simulink model "BMSModel"  (R2024b)
settings: Solver=VariableStepAuto  StartTime=0.0  StopTime=+inf  RelTol=1e-3
undefined symbols (not defined in the file): balancingRelaxationTime, balancingTime, ChargerParRes, ChargerRes, ChargingCurrentRequest, DeltaVTargetMin, DrivingCurrentRqst, InverterParRes, InverterRes, OpenCircuitInjection, PassiveBalancingRes, ShortCircuitInjection, sigbuilder_block, SystemState, SystemState_t
libraries: fl_lib x325, nesl_utility x223, batteryecm_lib x30, ee_lib x30
(part 17 of 33)

== system BMSModel/Electric Vehicul Model/HV Battery/Battery Pack/Battery Model1/Battery_Model/Cell3 ==
  ShortFault : Inport  OutDataTypeStr=boolean
  OpenFault : Inport  Port=2  OutDataTypeStr=boolean
  Battery (Table-Based)7 : batteryecm_lib/Battery (Table-Based)  PartSpecification="{\"DatasetId\":\"Panasonic|NCR18650BD|simscape.battery.batteryTableBased\",\"DisplayName\":\"Panasonic:NCR18650BD\"}"  SOC_vec=[0 0.01 0.02 0.03 0.04 0.05 0.06 0.07 0.08 0.09 0.1 0.11 0.12 0.13 0.14 0.15 0.16 0.17 0.18 0.19 0.2 0.21 0.22 0.23 0.24 0.25 0.26 0.27 0.28 0.29 0.3 0.31 0.32 0.33 0.34 0.35 0.36 0.37 0.38 0.39 0.4 0.41 0.42 0.43 0.44 0.45 0.46 0.47 0.48 0.49 0.5 0.51 0.52 0.53 0.54 0.55 0.56 0.57 0.58 0.59 0.6 0.61 0.62 0.63 0.64 0.65 0.66 0.67 0.68 0.69 0.7 0.71 0.72 0.73 0.74 0.75 0.76 0.77 0.78 0.79 0.8 0.81 0.82 0.83 0.84 0.85 0.86 0.87 0.88 0.89 0.9 0.91 0.92 0.93 0.94 0.95 0.96 0.97 0.98 0.99 1]  T_dependence=simscape.enum.tablebattery.temperature_dependence.yes  prm_dir=simscape.enum.tablebattery.prm_dir.noCurrentDirectionality  T_vec=[-10 0 25 40 60] degC  V0_mat=[3.01162183959268 3.01162183959268 3.01162183959268 3.01162183959268 3.01162183959268;3.08575650269622 3.08575650269622 3.08575650269622 3.08575650269622 3.08575650269622;3.14177499163339 3.14177499163339 3.14177499163339 3.14177499163339 3.14177499163339;3.18631823552003 3.18631823552003 3.18631823552003 3.18631823552003 3.18631823552003;3.22334954767724 3.22334954767724 3.22334954767724 3.22334954767724 3.22334954767724;3.25390136234099 3.25390136234099 3.25390136234099 3.25390136234099 3.25390136234099;3.27878869705109 3.27878869705109 3.27878869705109 3.27878869705109 3.27878869705109;3.30070350874259 3.30070350874259 3.30070350874259 3.30070350874259 3.30070350874259;3.32118523630503 3.32118523630503 3.32118523630503 3.32118523630503 3.32118523630503;3.33873222862982 3.33873222862982 3.33873222862982 3.33873222862982 3.33873222862982;3.35681278765286 3.35681278765286 3.35681278765286 3.35681278765286 3.35681278765286;3.3727103181478 3.3727103181478 3.3727103181478 3.3727103181478 3.3727103181478;3.38793252475269 3.38793252475269 3.38793252475269 3.38793252475269 3.38793252475269;3.40246133852855 3.40246133852855 3.40246133852855 3.40246133852855 3.40246133852855;3.41323877471787 3.41323877471787 3.41323877471787 3.41323877471787 3.41323877471787;3.42274053961048 3.42274053961048 3.42274053961048 3.42274053961048 3.42274053961048;3.43230513033606 3.43230513033606 3.43230513033606 3.43230513033606 3.43230513033606;3.44127066639713 3.44127066639713 3.44127066639713 3.44127066639713 3.44127066639713;3.45046092538443 3.45046092538443 3.45046092538443 3.45046092538443 3.45046092538443;3.46050021597496 3.46050021597496 3.46050021597496 3.46050021597496 3.46050021597496;3.47059444208772 3.47059444208772 3.47059444208772 3.47059444208772 3.47059444208772;3.47754582201769 3.47754582201769 3.47754582201769 3.47754582201769 3.47754582201769;3.48403887676592 3.48403887676592 3.48403887676592 3.48403887676592 3.48403887676592;3.49083263175798 3.49083263175798 3.49083263175798 3.49083263175798 3.49083263175798;3.49762638675004 3.49762638675004 3.49762638675004 3.49762638675004 3.49762638675004;3.50414427848956 3.50414427848956 3.50414427848956 3.50414427848956 3.50414427848956;3.51006189737342 3.51006189737342 3.51006189737342 3.51006189737342 3.51006189737342;3.51650779121088 3.51650779121088 3.51650779121088 3.51650779121088 3.51650779121088;3.52300031605604 3.52300031605604 3.52300031605604 3.52300031605604 3.52300031605604;3.5294928409012 3.5294928409012 3.5294928409012 3.5294928409012 3.5294928409012;3.53574150484027 3.53574150484027 3.53574150484027 3.53574150484027 3.53574150484027;3.54126505716736 3.54126505716736 3.54126505716736 3.54126505716736 3.54126505716736;3.54599126111241 3.54599126111241 3.54599126111241 3.54599126111241 3.54599126111241;3.55084210438451 3.55084210438451 3.55084210438451 3.55084210438451 3.55084210438451;3.55569196094666 3.55569196094666 3.55569196094666 3.55569196094666 3.55569196094666;3.56052372392349 3.56052372392349 3.56052372392349 3.56052372392349 3.56052372392349;3.56532059653064 3.56532059653064 3.56532059653064 3.56532059653064 3.56532059653064;3.56979945432131 3.56979945432131 3.56979945432131 3.56979945432131 3.56979945432131;3.57444121267711 3.57444121267711 3.57444121267711 3.57444121267711 3.57444121267711;3.57904303346503 3.57904303346503 3.57904303346503 3.57904303346503 3.57904303346503;3.58364485425295 3.58364485425295 3.58364485425295 3.58364485425295 3.58364485425295;3.58840361874736 3.58840361874736 3.58840361874736 3.58840361874736 3.58840361874736;3.5931676308725 3.5931676308725 3.5931676308725 3.5931676308725 3.5931676308725;3.59914045642067 3.59914045642067 3.59914045642067 3.59914045642067 3.59914045642067;3.60536834334135 3.60536834334135 3.60536834334135 3.60536834334135 3.60536834334135;3.61166009904557 3.61166009904557 3.61166009904557 3.61166009904557 3.61166009904557;3.61797346412467 3.61797346412467 3.61797346412467 3.61797346412467 3.61797346412467;3.62428682920377 ...(+4538 chars)] V  V0_vec=[3.01162183959268 3.08575650269622 3.14177499163339 3.18631823552003 3.22334954767724 3.25390136234099 3.27878869705109 3.30070350874259 3.32118523630503 3.33873222862982 3.35681278765286 3.3727103181478 3.38793252475269 3.40246133852855 3.41323877471787 3.42274053961048 3.43230513033606 3.44127066639713 3.45046092538443 3.46050021597496 3.47059444208772 3.47754582201769 3.48403887676592 3.49083263175798 3.49762638675004 3.50414427848956 3.51006189737342 3.51650779121088 3.52300031605604 3.5294928409012 3.53574150484027 3.54126505716736 3.54599126111241 3.55084210438451 3.55569196094666 3.56052372392349 3.56532059653064 3.56979945432131 3.57444121267711 3.57904303346503 3.58364485425295 3.58840361874736 3.5931676308725 3.59914045642067 3.60536834334135 3.61166009904557 3.61797346412467 3.62428682920377 3.63060019428287 3.63762035332317 3.64493091713641 3.65193784155324 3.65894476597006 3.66595169038689 3.67346631195551 3.68205597286888 3.69093403907072 3.69981210527255 3.70876940774879 3.71776352136614 3.72686785650522 3.73538877817079 3.74389655120326 3.7524031927436 3.76046489817023 3.76888762106825 3.7779024493972 3.78684332393235 3.79578419846749 3.80453642296868 3.81235798589345 3.82032795054978 3.82829600796219 3.83626406537459 3.84436267545785 3.8525073311682 3.86057855536852 3.86864977956884 3.87672100376915 3.8849361052788 3.89310639828889 3.90173837211624 3.91095173577988 3.92144530461468 3.9324037776784 3.94373568413836 3.95558619350771 3.96743304428185 3.9792002957498 3.99089798499064 4.00334195302942 4.01527114968024 4.0272843604214 4.03909414300309 4.04916289177822 4.05870791988182 4.06775178122738 4.07651000374835 4.08584401267932 4.09484130348047 4.10452854006648] V  V_range=[0, inf] V  R0_mat=[0.169226664942506 0.167163906452554 0.108383589577359 0.166496905009762 0.167551697989061;0.170828416476837 0.165751444040606 0.104276452250662 0.121215639388209 0.0793876425776906;0.169530739150659 0.158468431589049 0.0975073630060558 0.0973078843610968 0.0561405476976999;0.164751308135469 0.151517246065733 0.0937501268832215 0.0836197941247371 0.0456130421507902;0.161623504322352 0.145871801527593 0.0893819468578971 0.0721103823007927 0.0431619821725486;0.158185801688513 0.140686706979324 0.0854644713676261 0.0704282437680971 0.0421646037454825;0.154847008399576 0.135091432531267 0.08175755280038 0.0668766399041617 0.0396839944792877;0.151241736581272 0.129916346607537 0.0789657156023764 0.0623440053410213 0.0423144995660151;0.149319253943555 0.125715735974038 0.0763700410896046 0.0625490036389012 0.0444720395936461;0.147647439390094 0.12097131496664 0.074350005039498 0.0625868128655119 0.0460556489919985;0.146951059448331 0.118987805825618 0.0728433546029721 0.0628007167120074 0.0489709240971276;0.145717636926329 0.116283825223175 0.0716898267238281 0.0622941490970829 0.0511657277408373;0.144477070611268 0.114422945778514 0.0709947524858036 0.062440194574166 0.0531376522129154;0.143676208839892 0.113080299822462 0.0701675330798177 0.0632439744628587 0.0547714142497681;0.14163726865712 0.110499575455016 0.0687296636498536 0.0628096759401558 0.0549419374047933;0.139177314845068 0.107543098246653 0.0673712307537146 0.0619543637881734 0.0546914469305389;0.137590624546326 0.106293961693032 0.0662158126567781 0.0611197862345337 0.0544616910546272;0.135941778352897 0.104847117329339 0.0650205672726794 0.0601287634630414 0.054034227368643;0.134367098141725 0.103474438947903 0.0638581443118004 0.0597901127221725 0.0535283975759592;0.13307262638046 0.102457131174024 0.0632667622677959 0.0597316704312107 0.0532367466498601;0.132132723422297 0.101972409844216 0.0628323749302967 0.0596913586756406 0.0529632262591526;0.131094168008448 0.100450445549793 0.0619127995096658 0.058613803955455 0.0516524629038298;0.129904350158379 0.0987772188191482 0.0610362875653994 0.0575678584365115 0.0504293845103144;0.128813773312875 0.0972032330930701 0.0603620203223803 0.0567011683159597 0.0497501063026246;0.127723196467371 0.0960864247779599 0.0596877530793612 0.0558344781954072 0.049070828094934;0.126541575644132 0.0955051141824603 0.0589554578065768 0.0548767440971203 0.0483005059095089;0.125161844967536 0.0947256937336041 0.0582121279560435 0.0537209001454771 0.0473320738707274;0.124441622691283 0.0941206214542552 0.0577538001128378 0.0530375970606514 0.0465379900014533;0.123787439191075 0.0935309389464247 0.0573051863053598 0.0528219943808646 0.0460644521538233;0.123133255690868 0.0931004014618328 0.0568565724978822 0.052606391701078 0.0457423549094267;0.122398590043435 0.0926174478301112 0.0563566623767882 0.0523103068740658 0.0453397755178046;0.121424613632972 0.0918951834353595 0.0557889650711299 0.0517749112840235 0.0446978853631523;0.12018748594131 0.0909097677594091 0.0551527157042778 0.0509763644127827 0.0437928439273016;0.118892784708147 0.0899654871748907 0.0546601636283966 0.0502189526329738 0.0429623528682206;0.117592838262263 0.0890660124715378 0.0541676729953156 0.0494654301282659 0.0426851077795709;0.116286920336072 0.0881633432998133 0.0536763090566326 0.0487947175037582 0.042401891210615;0.11496948743639 0.0872491591545975 0.0531776059040762 0.0481124899057588 0.0421071596681674;0.113547099151729 0.0862300196244025 0.0526119236645951 0.0473253069227807 0.0417074727407412;0.112178473429822 0.0852646426569612 0.0520712300263533 0.0465918865025558 0.0413615483760683;0.110796666992441 0.0842860849740457 0.0515036752143963 0.045845285366857 0.0410024432959212;0.109615209979064 0.0832959889625606 0.0509361204024386 0.0450986842311574 0.0401864650192761;0.108594985808955 0.0823524881802858 0.050358792646556 0.0444566840657222 0.0392008538728351;0.107576493530178 0.0814107192893429 0.0497811381187243 0.0441001730364305 0.0382169746177263 ...(+5407 chars)] Ohm  R0_vec=[0.108383589577359 0.104276452250662 0.0975073630060558 0.0937501268832215 0.0893819468578971 0.0854644713676261 0.08175755280038 0.0789657156023764 0.0763700410896046 0.074350005039498 0.0728433546029721 0.0716898267238281 0.0709947524858036 0.0701675330798177 0.0687296636498536 0.0673712307537146 0.0662158126567781 0.0650205672726794 0.0638581443118004 0.0632667622677959 0.0628323749302967 0.0619127995096658 0.0610362875653994 0.0603620203223803 0.0596877530793612 0.0589554578065768 0.0582121279560435 0.0577538001128378 0.0573051863053598 0.0568565724978822 0.0563566623767882 0.0557889650711299 0.0551527157042778 0.0546601636283966 0.0541676729953156 0.0536763090566326 0.0531776059040762 0.0526119236645951 0.0520712300263533 0.0515036752143963 0.0509361204024386 0.050358792646556 0.0497811381187243 0.0494179007317464 0.0491061102548996 0.0488178197046288 0.0485440631684005 0.0482703066321729 0.0479965500959444 0.04783814754709 0.0477067926990279 0.0473712163371039 0.0470356399751792 0.0467000636132553 0.046431066764779 0.0463210072143651 0.0462716139198204 0.0462222206252753 0.0462261199933534 0.0462489440940044 0.0463125045945071 0.046258596645442 0.0462019228721321 0.0461444880808494 0.0458836890808223 0.04572086669122 0.0456935258730667 0.0456707901856076 0.0456480544981478 0.0455856361429723 0.0453236882021849 0.0450859495122444 0.0448483295871051 0.0446107096619657 0.0444608967355151 0.0443618965142366 0.0442474499230246 0.0441330033318128 0.0440185567406007 0.0437935866730952 0.0434800673484185 0.0432636629093245 0.0431695542324038 0.0433145404237094 0.0436166512557128 0.0439115402259747 0.0443155176840517 0.0447170344474344 0.0451426585495349 0.0455784262459232 0.0461653340876939 0.046366693627792 0.0465478472510504 0.046677433375216 0.0460938543815088 0.0452312262852973 0.044216043184874 0.0431407757562075 0.0413658805730101 0.0384867561999347 0.0332622873381256] Ohm  R0_dis_mat=[0.03 0.015 0.002;0.04 0.017 0.008;0.039 0.012 0.006;0.027 0.013 0.021] % Parameter not set Ohm  R0_dis_vec=[0.015 0.017 0.012 0.013] % Parameter not set Ohm  R0_ch_mat=[0.03 0.015 0.002;0.04 0.017 0.008;0.039 0.012 0.006;0.027 0.013 0.021] % Parameter not set Ohm  R0_ch_vec=[0.015 0.017 0.012 0.013] % Parameter not set Ohm  AH=[2.68349638863606 2.78552026614936 3.03732766876938 3.13981189304305 3.15345135361432] hr*A  prm_leak=simscape.enum.tablebattery.prm_leak.disabled  Rleak_vec=[8000 7000 6000] % Parameter not set Ohm  Rleak=7000 % Parameter not set Ohm  extrapolation_option=simscape.enum.extrapolation.linear  SOC_port=simscape.enum.tablebattery.enable.yes  prm_dyn=simscape.enum.tablebattery.prm_dyn.off  R1_mat=[0.089 0.076 0.01;0.042 0.022 0.099;0.019 0.007 0.002;0.051 0.043 0.029] % Parameter not set Ohm  R1_vec=[0.076 0.022 0.007 0.043] % Parameter not set Ohm  tau1_mat=[44 148 235;93 110 1000;19 27 133;0.5 22 3] % Parameter not set s  tau1_vec=[148 110 27 22] % Parameter not set s  R2_mat=[0.014 0.382 0.407;0.028 0.006 0.007;0.014 0.007 0.006;0.333 0.956 0.912] % Parameter not set Ohm  R2_vec=[0.382 0.006 0.007 0.956] % Parameter not set Ohm  tau2_mat=[1 44 5644;11 24 506;2 14 330;3310 13419 30216] % Parameter not set s  tau2_vec=[44 24 14 13419] % Parameter not set s  R3_mat=[0.014 0.382 0.407;0.028 0.006 0.007;0.014 0.007 0.006;0.333 0.956 0.912] % Parameter not set Ohm  R3_vec=[0.382 0.006 0.007 0.956] % Parameter not set Ohm  tau3_mat=[1 44 5644;11 24 506;2 14 330;3310 13419 30216] % Parameter not set s  tau3_vec=[44 24 14 13419] % Parameter not set s  R4_mat=[0.014 0.382 0.407;0.028 0.006 0.007;0.014 0.007 0.006;0.333 0.956 0.912] % Parameter not set Ohm  R4_vec=[0.382 0.006 0.007 0.956] % Parameter not set Ohm  tau4_mat=[1 44 5644;11 24 506;2 14 330;3310 13419 30216] % Parameter not set s  tau4_vec=[44 24 14 13419] % Parameter not set s  R5_mat=[0.014 0.382 0.407;0.028 0.006 0.007;0.014 0.007 0.006;0.333 0.956 0.912] % Parameter not set Ohm  R5_vec=[0.382 0.006 0.007 0.956] % Parameter not set Ohm  tau5_mat=[1 44 5644;11 24 506;2 14 330;3310 13419 30216] % Parameter not set s  tau5_vec=[44 24 14 13419] % Parameter not set s  prm_fade=simscape.enum.tablebattery.prm_fade.tableN  N0=100 % Parameter not set  dV0=0 % Parameter not set  dR0=0 % Parameter not set  dAH=0 % Parameter not set  dRleak=0 % Parameter not set  dR1=0 % Parameter not set  dR2=0 % Parameter not set  dR3=0 % Parameter not set  dR4=0 % Parameter not set  dR5=0 % Parameter not set  N0vec=[1 5 9 13 17 21 25 29 33 37 41 45 49 53 57 61 65 69 73 77 81 85 89 93 97 101 105 109 113 117 121 125 129 133 137 141 145 149 153 157 161 165 169 173 177 181 185 189 193 197 201 205 209 213 217 221 225 229 233 237 241 245 249 253 257 261 265 269 273 277 281 285 289 293 297 301 305 309 313 317]  Tfadevec=[298.15 323.15] % Parameter not set K  dV0vec=[0 -0.202976020343784 -0.3923769038231 -0.473851165498915 -0.557677193828717 -0.641503222158497 -0.722694953681098 -0.78693536781202 -0.84923483782873 -0.91204079498593 -0.976372086795922 -1.04070337860591 -1.09651419150015 -1.13609671747983 -1.17677806371868 -1.21745940995752 -1.25814075619637 -1.29882210243524 -1.33950344867411 -1.38190179312493 -1.42481526081378 -1.46425241362882 -1.5010921128618 -1.53793181209476 -1.57477151132773 -1.61462185034629 -1.65783167194698 -1.70104149354772 -1.74425131514844 -1.78746113674918 -1.83079973190849 -1.87421420391064 -1.91824367282699 -1.96240597622001 -2.00656827961306 -2.05073058300608 -2.09489288639913 -2.13905518979217 -2.17980859972762 -2.21447670676208 -2.24914481379657 -2.28381292083105 -2.3184810278655 -2.35314913489998 -2.38781724193444 -2.42248534896891 -2.45715345600337 -2.49296850346706 -2.52769329927103 -2.561744919227 -2.59579653918297 -2.62984815913894 -2.66389977909491 -2.69795139905088 -2.73200301900684 -2.76605463896281 -2.79820594865531 -2.82900596880459 -2.85607497867955 -2.88314398855452 -2.9102129984295 -2.93728200830447 -2.96435101817944 -2.99142002805441 -3.01848903792938 -3.04532930155381 -3.07028784008487 -3.09529445491126 -3.12030106973765 -3.14530768456405 -3.17031429939046 -3.19532091421685 -3.22227871749008 -3.25169561441252 -3.28111251133495 -3.31052940825736 -3.3399463051798 -3.36956112962439 -3.39917701648019 -3.42879290333599]  dR0vec=[0;0;0;0;0;0;0;0;0;0;0;0;0;0;0;0;0;0;0;0;0;0;0;0;0;0;0;0;0;0;0;0;0;0;0;0;0;0;0;0;0;0;0;0;0;0;0;0;0;0;0;0;0;0;0;0;0;0;0;0;0;0;0;0;0;0;0;0;0;0;0;0;0;0;0;0;0;0;0;0] % Parameter not set  dAHvec=[0 -0.860019120059007 -1.71332421702079 -2.08194002639565 -2.45055583577056 -2.81917164514544 -3.18778745452034 -3.48884924256567 -3.78081482359822 -4.07278040463078 -4.36474598566332 -4.65671156669588 -4.91000721732096 -5.08494410055826 -5.25988098379556 -5.43481786703286 -5.60975475027016 -5.78469163350746 -5.95962851674477 -6.13456539998206 -6.30950228321938 -6.47026795816407 -6.62044510557951 -6.77062225299495 -6.92079940041038 -7.07097654782583 -7.22115369524125 -7.3713308426567 -7.52150799007212 -7.67168513748756 -7.8223098416152 -7.97319825819041 -8.12408667476563 -8.27497509134084 -8.42586350791606 -8.57675192449127 -8.72764034106648 -8.8785287576417 -9.01789522921441 -9.13717923376072 -9.25646323830702 -9.37574724285333 -9.49503124739961 -9.61431525194592 -9.73359925649221 -9.85288326103849 -9.9721672655848 -10.0914512701311 -10.2059878190689 -10.3183039593139 -10.430620099559 -10.542936239804 -10.655252380049 -10.7675685202941 -10.8798846605391 -10.9922008007841 -11.1045169410292 -11.2125891928512 -11.3075699375911 -11.402550682331 -11.4975314270709 -11.5925121718108 -11.6874929165507 -11.7824736612906 -11.8774544060305 -11.9716325174515 -12.058857953676 -12.1460833899004 -12.2333088261248 -12.3205342623493 -12.4077596985737 -12.4949851347981 -12.5890165007581 -12.6916254178083 -12.7942343348584 -12.8968432519086 -12.9994521689588 -13.102061086009 -13.2046700030592 -13.3072789201093]  dRleakvec=[0 0 0] % Parameter not set  dR1vec=[0 0 0] % Parameter not set  dR2vec=[0 0 0] % Parameter not set  dR3vec=[0 0 0] % Parameter not set  dR4vec=[0 0 0] % Parameter not set  dR5vec=[0 0 0] % Parameter not set  dV0mat=[0 0;0 0;0 0] % Parameter not set  dR0mat=[0 0;0 0;0 0] % Parameter not set  dAHmat=[0 0;0 0;0 0] % Parameter not set  dRleakmat=[0 0;0 0;0 0] % Parameter not set  dR1mat=[0 0;0 0;0 0] % Parameter not set  dR2mat=[0 0;0 0;0 0] % Parameter not set  dR3mat=[0 0;0 0;0 0] % Parameter not set  dR4mat=[0 0;0 0;0 0] % Parameter not set  dR5mat=[0 0;0 0;0 0] % Parameter not set  prm_age_modeling=simscape.enum.tablebattery.prm_age_modeling.equation  storage_dt_age_vec=0 % Parameter not set d  storage_T_age_vec=273 % Parameter not set K  prm_age_OCV=simscape.enum.tablebattery.prm_age_OCV.SOC  OCV_age=0.9 % Parameter not set  SOC_age=0.6 % Parameter not set  prm_age_resistance=simscape.enum.tablebattery.prm_age.disabled  OCV_scaling_age_resistance=2213400  OCV_offset_age_resistance=1632000  temp_exp_age_resistance=0.515833569 V  t_exp_age_resistance=0.75  T_age_vec_resistance=[273.15 298.15 323.15] K  dt_age_vec_resistance=[90 180 270 360] d  dR0_age_vec=[0 0 0] % Parameter not set  t_meas_dR0_age=100 % Parameter not set d  dR0_age_mat=[0 0 0;0 0 0;0 0 0;0 0 0] % Parameter not set  prm_age_capacity=simscape.enum.tablebattery.prm_age.disabled  OCV_scaling_age_capacity=1.5097e07  OCV_offset_age_capacity=8.3625e06  temp_exp_age_capacity=0.6011 V  t_exp_age_capacity=0.75  T_age_vec_capacity=[273.15, 298.15, 323.15] K  dt_age_vec_capacity=[0, 90, 180, 270] d  dAH_age_vec=[0, 0, 0]  t_meas_dAH_age=100 d  dAH_age_mat=[0, 0, 0; 0, 0, 0; 0, 0, 0; 0, 0, 0]  thermal_port=simscape.enum.thermaleffects.model  temperature=298.15 K  thermal_mass=CellThermalMass J/K  i_specify=off  i_priority=None  i=0 A  i_nominal_specify=off  i_nominal_value=1  i_nominal_unit=A  v_specify=off  v_priority=None  v=0 V  v_nominal_specify=off  v_nominal_value=1  v_nominal_unit=V  stateOfCharge_specify=on  stateOfCharge_priority=High  stateOfCharge=1  stateOfCharge_nominal_specify=off  stateOfCharge_nominal_value=1  stateOfCharge_nominal_unit=1  num_cycles_specify=off  num_cycles_priority=High  num_cycles=0  num_cycles_nominal_specify=off  num_cycles_nominal_value=1  num_cycles_nominal_unit=1  cell_temperature_specify=on  cell_temperature_priority=High  cell_temperature=298.15 K  cell_temperature_nominal_specify=off  cell_temperature_nominal_value=1  cell_temperature_nominal_unit=K
      settings: AttributesFormatString=Panasonic:NCR18650BD
  Cast To Double : DataTypeConversion  OutDataTypeStr=double  SaturateOnIntegerOverflow=off  RndMeth=Floor
  Cast To Double1 : DataTypeConversion  OutDataTypeStr=double  SaturateOnIntegerOverflow=off  RndMeth=Floor
  Conductive Heat Transfer1 : fl_lib/Thermal/Thermal Elements/Conductive Heat Transfer  thermal_type=foundation.enum.ConstantVariableTabulated.Constant  wall_geometry=foundation.enum.ConductionWallGeometry.Planar  area=Cell2CellConductiveArea m^2  thickness=Cell2CellConductiveThickness mm  d_in=0.05 m  d_out=0.1 m  L=1 m  th_cond_min=10 W/(K*m)  th_cond=Cell2CellConductiveThermConduct W/(m*K)  th_cond_TLU=[413, 401, 392, 383, 371, 357, 342] W/(K*m)  temp_TLU=[200, 273, 400, 600, 800, 1000, 1200] K  T_specify=off  T_priority=None  T=0 K  T_nominal_specify=off  T_nominal_value=1  T_nominal_unit=K  Q_specify=off  Q_priority=None  Q=0 W  Q_nominal_specify=off  Q_nominal_value=1  Q_nominal_unit=W
  Convective Heat Transfer1 : fl_lib/Thermal/Thermal Elements/Convective Heat Transfer  thermal_type=foundation.enum.constant_variable.constant  area=CellArea m^2  heat_tr_coeff=CellConvectiveCoeff W/(m^2 * K)  min_heat_tr_coeff=1e-3 W/(K*m^2)  T_specify=off  T_priority=None  T=0 K  T_nominal_specify=off  T_nominal_value=1  T_nominal_unit=K  Q_specify=off  Q_priority=None  Q=0 W  Q_nominal_specify=off  Q_nominal_value=1  Q_nominal_unit=W
  Current Sensor1 : fl_lib/Electrical/Electrical Sensors/Current Sensor
  OpenSwitch1 : fl_lib/Electrical/Electrical Elements/Switch  R_closed=1e-12 Ohm  G_open=1e-10 1/Ohm  Threshold=0.5  i_specify=off  i_priority=None  i=0 A  i_nominal_specify=off  i_nominal_value=1  i_nominal_unit=A  v_specify=off  v_priority=None  v=0 V  v_nominal_specify=off  v_nominal_value=1  v_nominal_unit=V
  PS-Simulink Converter1 : nesl_utility/PS-Simulink Converter  PhysicalDomain=network_engine_domain  SubClassName=ps_output  LeftPortType=input  RightPortType=output  PseudoPeriodic=off  Frequencies=[]  VectorFormat=1-D array  Unit=inherit  AffineConversion=off
  PS-Simulink Converter2 : nesl_utility/PS-Simulink Converter  PhysicalDomain=network_engine_domain  SubClassName=ps_output  LeftPortType=input  RightPortType=output  PseudoPeriodic=off  Frequencies=[]  VectorFormat=1-D array  Unit=inherit  AffineConversion=off
  PS-Simulink Converter3 : nesl_utility/PS-Simulink Converter  PhysicalDomain=network_engine_domain  SubClassName=ps_output  LeftPortType=input  RightPortType=output  PseudoPeriodic=off  Frequencies=[]  VectorFormat=1-D array  Unit=inherit  AffineConversion=off
  ShortSwitch1 : fl_lib/Electrical/Electrical Elements/Switch  R_closed=1e-10 Ohm  G_open=1e-10 1/Ohm  Threshold=0.5  i_specify=off  i_priority=None  i=0 A  i_nominal_specify=off  i_nominal_value=1  i_nominal_unit=A  v_specify=off  v_priority=None  v=0 V  v_nominal_specify=off  v_nominal_value=1  v_nominal_unit=V
  Simulink-PS Converter : nesl_utility/Simulink-PS Converter  PhysicalDomain=network_engine_domain  SubClassName=ps_input  LeftPortType=input  RightPortType=output  PseudoPeriodic=off  Frequencies=[]  NoiseDistribution=none  NoiseParameters=[]  Unit=1  AffineConversion=off  FilteringAndDerivatives=provide  SimscapeFilterOrder=1  InputFilterTimeConstant=0.001  UdotUserProvided=0
  Simulink-PS Converter1 : nesl_utility/Simulink-PS Converter  PhysicalDomain=network_engine_domain  SubClassName=ps_input  LeftPortType=input  RightPortType=output  PseudoPeriodic=off  Frequencies=[]  NoiseDistribution=none  NoiseParameters=[]  Unit=1  AffineConversion=off  FilteringAndDerivatives=provide  SimscapeFilterOrder=1  InputFilterTimeConstant=0.001  UdotUserProvided=0
  Temperature Sensor1 : fl_lib/Thermal/Thermal Sensors/Temperature Sensor  temperature_measure=foundation.enum.MeasurementReference.difference
  Thermal Reference1 : fl_lib/Thermal/Thermal Elements/Thermal Reference
  HeatIn : PMIOPort  Side=Left
  HeatOut : PMIOPort  Port=2  Side=Left
  V+ : PMIOPort  Port=3  Side=Right
  V- : PMIOPort  Port=4  Side=Right
  TCell : Outport
  RealSOC : Outport  Port=2
  Current : Outport  Port=3
  wires:
  OpenFault/1 -> Cast To Double1/1
  Cast To Double1/1 -> Simulink-PS Converter/1
  ShortFault/1 -> Cast To Double/1
  Cast To Double/1 -> Simulink-PS Converter1/1
  PS-Simulink Converter2/1 -> TCell/1
  PS-Simulink Converter1/1 -> RealSOC/1
  PS-Simulink Converter3/1 -> Current/1
  connections:
  OpenSwitch1/RConn2 -- ShortSwitch1/RConn2 -- V-/RConn1
  HeatOut/RConn1 -- Convective Heat Transfer1/RConn1 -- Temperature Sensor1/LConn1 -- Battery (Table-Based)7/RConn2
  V+/RConn1 -- Current Sensor1/RConn2 -- ShortSwitch1/LConn1
  Simulink-PS Converter1/RConn1 -- ShortSwitch1/RConn1
  Battery (Table-Based)7/LConn2 -- PS-Simulink Converter1/LConn1
  Thermal Reference1/LConn1 -- Temperature Sensor1/RConn1
  PS-Simulink Converter2/LConn1 -- Temperature Sensor1/RConn2
  OpenSwitch1/LConn1 -- Battery (Table-Based)7/RConn1
  PS-Simulink Converter3/LConn1 -- Current Sensor1/RConn1
  Battery (Table-Based)7/LConn1 -- Current Sensor1/LConn1
  Convective Heat Transfer1/LConn1 -- HeatIn/RConn1
  Simulink-PS Converter/RConn1 -- OpenSwitch1/RConn1
